simulink model "adc5g_test"  (Simulink 8.0)
settings: Solver=VariableStepDiscrete  SolverMode=SingleTasking  StartTime=0.0  StopTime=10000  RelTol=1e-3
libraries: xbsIndex_r4 x4240, dspsigops x32, casper_library_misc x32, simulink x2, xps_library x1
(part 18 of 29)

== system adc5g_test/hist_0/core_c/subhist0/control ==
  sync : Inport
  bin : Inport  Port=2
  count : Inport  Port=3
  Constant1 : xbsIndex_r4/Constant  const=0  gui_display_data_type=Fixed-point  arith_type=Unsigned  n_bits=46  bin_pt=0  preci_type=Single  exp_width=8  frac_width=24  explicit_period=on  period=1  equ=P=C  opselect=C  inp2=PCIN>>17  opr=+  inp1=P  carry=CIN  dbl_ovrd=off  block_type=constant
  Counter : xbsIndex_r4/Counter  cnt_type=Count Limited  cnt_to=255  operation=Up  start_count=0  cnt_by_val=1  arith_type=Unsigned  n_bits=8  bin_pt=0  load_pin=off  rst=on  en=off  explicit_period=on  period=1  dbl_ovrd=off  use_behavioral_HDL=off  implementation=Fabric  block_type=counter
  Delay17 : xbsIndex_r4/Delay  rst=off  infoeditControl=Selection of Reset will increase slice count due to use of real FFs and instead of SRLs  en=off  latency=7  dbl_ovrd=off  reg_retiming=off  block_type=delay
  Delay2 : xbsIndex_r4/Delay  rst=off  infoeditControl=Selection of Reset will increase slice count due to use of real FFs and instead of SRLs  en=off  latency=7  dbl_ovrd=off  reg_retiming=off  block_type=delay
  Delay3 : xbsIndex_r4/Delay  rst=off  infoeditControl=Selection of Reset will increase slice count due to use of real FFs and instead of SRLs  en=off  latency=7  dbl_ovrd=off  reg_retiming=off  block_type=delay
  Delay5 : xbsIndex_r4/Delay  rst=off  infoeditControl=Selection of Reset will increase slice count due to use of real FFs and instead of SRLs  en=off  latency=1  dbl_ovrd=off  reg_retiming=off  block_type=delay
  Inverter1 : xbsIndex_r4/Inverter  en=off  latency=0  dbl_ovrd=off  block_type=inv
  Logical : xbsIndex_r4/Logical  logical_function=AND  inputs=2  en=off  latency=0  precision=Full  arith_type=Unsigned  n_bits=16  bin_pt=0  align_bp=on  dbl_ovrd=off  block_type=logical
  Mux2 : xbsIndex_r4/Mux  inputs=2  en=off  latency=0  precision=Full  arith_type=Unsigned  n_bits=16  bin_pt=14  quantization=Truncate  overflow=Wrap  dbl_ovrd=off  block_type=mux
  edge_detect : Subsystem  AncestorBlock=casper_library_misc/edge_detect  mask(edge_detect){edge=Rising, polarity=Active High, x_in=[0 0.08 0.08 0.16 0.16 0.24 0.24 0.32 0.32 0.4], y_in=[-1 -1  1  1  1  1  1  1  1  1], x_out=[0.6 0.68 0.68 0.76 0.76 0.84 0.84 0.92 0.92 1], y_out=[-1 -1  1  1 -1 -1 -1 -1 -1 -1]}
      mask prompts: edge "Edge Type"; polarity "Output Polarity"; x_in "Input X Positions"; y_in "Input Y Positions"; x_out "Output X Positions"; y_out "Output Y Positions"
      mask help: Output is active for one-cycle after each edge of the specified type (i.e. rising, falling or both).
      mask init: same as adc5g_test/ramptest_0/posedge
      settings: AttributesFormatString=|
      | rising edges
      | active high
      mask Help: Output is active for one-cycle after each edge of the specified type (i.e. rising, falling, or both).  Latency is zero.
      mask TypeOptions edge: Rising | Falling | Both
      mask TypeOptions polarity: Active High | Active Low
      mask Display:
      | plot(x_in_num,0.5+y_in_num*0.2);
      | plot(x_out_num,0.5+y_out_num*0.2);
  pulse_ext : casper_library_misc/pulse_ext  pulse_len=256
  addra : Outport
  dina : Outport  Port=2
  wea : Outport  Port=3
  addrb : Outport  Port=4
  dinb : Outport  Port=5
  web : Outport  Port=6
  wires:
  sync/1 -> Delay17/1
  Mux2/1 -> addra/1, Delay2/1
  pulse_ext/1 -> Mux2/1, wea/1, Inverter1/1
  Delay5/1 -> Mux2/2
  Delay2/1 -> addrb/1
  Constant1/1 -> dina/1
  Logical/1 -> web/1
  edge_detect/1(out) -> pulse_ext/1, Counter/1
  Counter/1 -> Mux2/3
  Delay3/1 -> Logical/2
  Inverter1/1 -> Logical/1, Delay3/1
  Delay17/1 -> edge_detect/1(in)
  bin/1 -> Delay5/1
  count/1 -> dinb/1

== system adc5g_test/hist_0/core_c/subhist0/precounter ==
  din : Inport
  Constant3 : xbsIndex_r4/Constant  const=1  gui_display_data_type=Fixed-point  arith_type=Unsigned  n_bits=1  bin_pt=0  preci_type=Single  exp_width=8  frac_width=24  explicit_period=on  period=1  equ=P=C  opselect=C  inp2=PCIN>>17  opr=+  inp1=P  carry=CIN  dbl_ovrd=off  block_type=constant
  Delay{1..7} (7 alike) : xbsIndex_r4/Delay  rst=off  infoeditControl=Selection of Reset will increase slice count due to use of real FFs and instead of SRLs  en=off  latency=1  dbl_ovrd=off  reg_retiming=off  block_type=delay
  Reinterpret{,1..6} (7 alike) : xbsIndex_r4/Reinterpret  force_arith_type=on  arith_type=Unsigned  force_bin_pt=off  bin_pt=0  block_type=reinterpret
  Relational{1..7} (7 alike) : xbsIndex_r4/Relational  mode=a=b  en=off  latency=0  dbl_ovrd=off  block_type=relational
  cnt{1..7} (7 alike) : xbsIndex_r4/AddSub  mode=Addition  use_carryin=off  use_carryout=off  en=off  latency=1  precision=Full  arith_type=Unsigned  n_bits=64  bin_pt=0  quantization=Truncate  overflow=Saturate  dbl_ovrd=off  use_behavioral_HDL=off  hw_selection=Fabric  pipelined=on  block_type=addsub
  sum : Outport
  wires:
  cnt1/1 -> cnt3/2
  Constant3/1 -> cnt2/1
  Relational1/1 -> Reinterpret/1
  Relational3/1 -> Reinterpret2/1
  Relational2/1 -> Reinterpret1/1
  cnt2/1 -> cnt3/1
  Reinterpret/1 -> cnt2/2
  Reinterpret2/1 -> cnt1/2
  Delay4/1 -> Relational3/1, Delay6/1
  Reinterpret1/1 -> cnt1/1
  Delay6/1 -> Relational2/1, Delay7/1
  Delay7/1 -> Relational1/1
  cnt5/1 -> cnt6/2
  Relational4/1 -> Reinterpret3/1
  Relational6/1 -> Reinterpret5/1
  Relational5/1 -> Reinterpret4/1
  cnt4/1 -> cnt6/1
  Reinterpret3/1 -> cnt4/2
  Reinterpret5/1 -> cnt5/2
  Reinterpret4/1 -> cnt5/1
  Relational7/1 -> Reinterpret6/1
  Reinterpret6/1 -> cnt4/1
  din/1 -> Relational5/2, Relational4/2, Relational7/2, Relational3/2, Relational2/2, Relational1/2, Relational6/2, Delay5/1
  Delay1/1 -> Relational7/1, Delay4/1
  Delay2/1 -> Relational4/1, Delay1/1
  Delay3/1 -> Relational5/1, Delay2/1
  Delay5/1 -> Relational6/1, Delay3/1
  cnt3/1 -> cnt7/1
  cnt6/1 -> cnt7/2
  cnt7/1 -> sum/1

== system adc5g_test/hist_0/core_c/subhist1/control ==
  sync : Inport
  bin : Inport  Port=2
  count : Inport  Port=3
  Constant1 : xbsIndex_r4/Constant  const=0  gui_display_data_type=Fixed-point  arith_type=Unsigned  n_bits=46  bin_pt=0  preci_type=Single  exp_width=8  frac_width=24  explicit_period=on  period=1  equ=P=C  opselect=C  inp2=PCIN>>17  opr=+  inp1=P  carry=CIN  dbl_ovrd=off  block_type=constant
  Counter : xbsIndex_r4/Counter  cnt_type=Count Limited  cnt_to=255  operation=Up  start_count=0  cnt_by_val=1  arith_type=Unsigned  n_bits=8  bin_pt=0  load_pin=off  rst=on  en=off  explicit_period=on  period=1  dbl_ovrd=off  use_behavioral_HDL=off  implementation=Fabric  block_type=counter
  Delay17 : xbsIndex_r4/Delay  rst=off  infoeditControl=Selection of Reset will increase slice count due to use of real FFs and instead of SRLs  en=off  latency=7  dbl_ovrd=off  reg_retiming=off  block_type=delay
  Delay2 : xbsIndex_r4/Delay  rst=off  infoeditControl=Selection of Reset will increase slice count due to use of real FFs and instead of SRLs  en=off  latency=7  dbl_ovrd=off  reg_retiming=off  block_type=delay
  Delay3 : xbsIndex_r4/Delay  rst=off  infoeditControl=Selection of Reset will increase slice count due to use of real FFs and instead of SRLs  en=off  latency=7  dbl_ovrd=off  reg_retiming=off  block_type=delay
  Delay5 : xbsIndex_r4/Delay  rst=off  infoeditControl=Selection of Reset will increase slice count due to use of real FFs and instead of SRLs  en=off  latency=1  dbl_ovrd=off  reg_retiming=off  block_type=delay
  Inverter1 : xbsIndex_r4/Inverter  en=off  latency=0  dbl_ovrd=off  block_type=inv
  Logical : xbsIndex_r4/Logical  logical_function=AND  inputs=2  en=off  latency=0  precision=Full  arith_type=Unsigned  n_bits=16  bin_pt=0  align_bp=on  dbl_ovrd=off  block_type=logical
  Mux2 : xbsIndex_r4/Mux  inputs=2  en=off  latency=0  precision=Full  arith_type=Unsigned  n_bits=16  bin_pt=14  quantization=Truncate  overflow=Wrap  dbl_ovrd=off  block_type=mux
  edge_detect : Subsystem  AncestorBlock=casper_library_misc/edge_detect  mask(edge_detect){edge=Rising, polarity=Active High, x_in=[0 0.08 0.08 0.16 0.16 0.24 0.24 0.32 0.32 0.4], y_in=[-1 -1  1  1  1  1  1  1  1  1], x_out=[0.6 0.68 0.68 0.76 0.76 0.84 0.84 0.92 0.92 1], y_out=[-1 -1  1  1 -1 -1 -1 -1 -1 -1]}
      mask prompts: edge "Edge Type"; polarity "Output Polarity"; x_in "Input X Positions"; y_in "Input Y Positions"; x_out "Output X Positions"; y_out "Output Y Positions"
      mask help: Output is active for one-cycle after each edge of the specified type (i.e. rising, falling or both).
      mask init: same as adc5g_test/ramptest_0/posedge
      settings: AttributesFormatString=|
      | rising edges
      | active high
      mask Help: Output is active for one-cycle after each edge of the specified type (i.e. rising, falling, or both).  Latency is zero.
      mask TypeOptions edge: Rising | Falling | Both
      mask TypeOptions polarity: Active High | Active Low
      mask Display:
      | plot(x_in_num,0.5+y_in_num*0.2);
      | plot(x_out_num,0.5+y_out_num*0.2);
  pulse_ext : casper_library_misc/pulse_ext  pulse_len=256
  addra : Outport
  dina : Outport  Port=2
  wea : Outport  Port=3
  addrb : Outport  Port=4
  dinb : Outport  Port=5
  web : Outport  Port=6
  wires:
  count/1 -> dinb/1
  bin/1 -> Delay5/1
  Delay17/1 -> edge_detect/1(in)
  Inverter1/1 -> Delay3/1, Logical/1
  Delay3/1 -> Logical/2
  Counter/1 -> Mux2/3
  edge_detect/1(out) -> Counter/1, pulse_ext/1
  Logical/1 -> web/1
  Constant1/1 -> dina/1
  Delay2/1 -> addrb/1
  Delay5/1 -> Mux2/2
  pulse_ext/1 -> Inverter1/1, wea/1, Mux2/1
  Mux2/1 -> Delay2/1, addra/1
  sync/1 -> Delay17/1

== system adc5g_test/hist_0/core_c/subhist1/precounter ==
  din : Inport
  Constant3 : xbsIndex_r4/Constant  const=1  gui_display_data_type=Fixed-point  arith_type=Unsigned  n_bits=1  bin_pt=0  preci_type=Single  exp_width=8  frac_width=24  explicit_period=on  period=1  equ=P=C  opselect=C  inp2=PCIN>>17  opr=+  inp1=P  carry=CIN  dbl_ovrd=off  block_type=constant
  Delay{1..7} (7 alike) : xbsIndex_r4/Delay  rst=off  infoeditControl=Selection of Reset will increase slice count due to use of real FFs and instead of SRLs  en=off  latency=1  dbl_ovrd=off  reg_retiming=off  block_type=delay
  Reinterpret{,1..6} (7 alike) : xbsIndex_r4/Reinterpret  force_arith_type=on  arith_type=Unsigned  force_bin_pt=off  bin_pt=0  block_type=reinterpret
  Relational{1..7} (7 alike) : xbsIndex_r4/Relational  mode=a=b  en=off  latency=0  dbl_ovrd=off  block_type=relational
  cnt{1..7} (7 alike) : xbsIndex_r4/AddSub  mode=Addition  use_carryin=off  use_carryout=off  en=off  latency=1  precision=Full  arith_type=Unsigned  n_bits=64  bin_pt=0  quantization=Truncate  overflow=Saturate  dbl_ovrd=off  use_behavioral_HDL=off  hw_selection=Fabric  pipelined=on  block_type=addsub
  sum : Outport
  wires:
  cnt7/1 -> sum/1
  cnt6/1 -> cnt7/2
  cnt3/1 -> cnt7/1
  Delay5/1 -> Delay3/1, Relational6/1
  Delay3/1 -> Delay2/1, Relational5/1
  Delay2/1 -> Delay1/1, Relational4/1
  Delay1/1 -> Delay4/1, Relational7/1
  din/1 -> Delay5/1, Relational6/2, Relational1/2, Relational2/2, Relational3/2, Relational7/2, Relational4/2, Relational5/2
  Reinterpret6/1 -> cnt4/1
  Relational7/1 -> Reinterpret6/1
  Reinterpret4/1 -> cnt5/1
  Reinterpret5/1 -> cnt5/2
  Reinterpret3/1 -> cnt4/2
  cnt4/1 -> cnt6/1
  Relational5/1 -> Reinterpret4/1
  Relational6/1 -> Reinterpret5/1
  Relational4/1 -> Reinterpret3/1
  cnt5/1 -> cnt6/2
  Delay7/1 -> Relational1/1
  Delay6/1 -> Delay7/1, Relational2/1
  Reinterpret1/1 -> cnt1/1
  Delay4/1 -> Delay6/1, Relational3/1
  Reinterpret2/1 -> cnt1/2
  Reinterpret/1 -> cnt2/2
  cnt2/1 -> cnt3/1
  Relational2/1 -> Reinterpret1/1
  Relational3/1 -> Reinterpret2/1
  Relational1/1 -> Reinterpret/1
  Constant3/1 -> cnt2/1
  cnt1/1 -> cnt3/2

== system adc5g_test/hist_0/core_c/subhist2/control ==
  sync : Inport
  bin : Inport  Port=2
  count : Inport  Port=3
  Constant1 : xbsIndex_r4/Constant  const=0  gui_display_data_type=Fixed-point  arith_type=Unsigned  n_bits=46  bin_pt=0  preci_type=Single  exp_width=8  frac_width=24  explicit_period=on  period=1  equ=P=C  opselect=C  inp2=PCIN>>17  opr=+  inp1=P  carry=CIN  dbl_ovrd=off  block_type=constant
  Counter : xbsIndex_r4/Counter  cnt_type=Count Limited  cnt_to=255  operation=Up  start_count=0  cnt_by_val=1  arith_type=Unsigned  n_bits=8  bin_pt=0  load_pin=off  rst=on  en=off  explicit_period=on  period=1  dbl_ovrd=off  use_behavioral_HDL=off  implementation=Fabric  block_type=counter
  Delay17 : xbsIndex_r4/Delay  rst=off  infoeditControl=Selection of Reset will increase slice count due to use of real FFs and instead of SRLs  en=off  latency=7  dbl_ovrd=off  reg_retiming=off  block_type=delay
  Delay2 : xbsIndex_r4/Delay  rst=off  infoeditControl=Selection of Reset will increase slice count due to use of real FFs and instead of SRLs  en=off  latency=7  dbl_ovrd=off  reg_retiming=off  block_type=delay
  Delay3 : xbsIndex_r4/Delay  rst=off  infoeditControl=Selection of Reset will increase slice count due to use of real FFs and instead of SRLs  en=off  latency=7  dbl_ovrd=off  reg_retiming=off  block_type=delay
  Delay5 : xbsIndex_r4/Delay  rst=off  infoeditControl=Selection of Reset will increase slice count due to use of real FFs and instead of SRLs  en=off  latency=1  dbl_ovrd=off  reg_retiming=off  block_type=delay
  Inverter1 : xbsIndex_r4/Inverter  en=off  latency=0  dbl_ovrd=off  block_type=inv
  Logical : xbsIndex_r4/Logical  logical_function=AND  inputs=2  en=off  latency=0  precision=Full  arith_type=Unsigned  n_bits=16  bin_pt=0  align_bp=on  dbl_ovrd=off  block_type=logical
  Mux2 : xbsIndex_r4/Mux  inputs=2  en=off  latency=0  precision=Full  arith_type=Unsigned  n_bits=16  bin_pt=14  quantization=Truncate  overflow=Wrap  dbl_ovrd=off  block_type=mux
  edge_detect : Subsystem  AncestorBlock=casper_library_misc/edge_detect  mask(edge_detect){edge=Rising, polarity=Active High, x_in=[0 0.08 0.08 0.16 0.16 0.24 0.24 0.32 0.32 0.4], y_in=[-1 -1  1  1  1  1  1  1  1  1], x_out=[0.6 0.68 0.68 0.76 0.76 0.84 0.84 0.92 0.92 1], y_out=[-1 -1  1  1 -1 -1 -1 -1 -1 -1]}
      mask prompts: edge "Edge Type"; polarity "Output Polarity"; x_in "Input X Positions"; y_in "Input Y Positions"; x_out "Output X Positions"; y_out "Output Y Positions"
      mask help: Output is active for one-cycle after each edge of the specified type (i.e. rising, falling or both).
      mask init: same as adc5g_test/ramptest_0/posedge
      settings: AttributesFormatString=|
      | rising edges
      | active high
      mask Help: Output is active for one-cycle after each edge of the specified type (i.e. rising, falling, or both).  Latency is zero.
      mask TypeOptions edge: Rising | Falling | Both
      mask TypeOptions polarity: Active High | Active Low
      mask Display:
      | plot(x_in_num,0.5+y_in_num*0.2);
      | plot(x_out_num,0.5+y_out_num*0.2);
  pulse_ext : casper_library_misc/pulse_ext  pulse_len=256
  addra : Outport
  dina : Outport  Port=2
  wea : Outport  Port=3
  addrb : Outport  Port=4
  dinb : Outport  Port=5
  web : Outport  Port=6
  wires:
  sync/1 -> Delay17/1
  Mux2/1 -> addra/1, Delay2/1
  pulse_ext/1 -> Mux2/1, wea/1, Inverter1/1
  Delay5/1 -> Mux2/2
  Delay2/1 -> addrb/1
  Constant1/1 -> dina/1
  Logical/1 -> web/1
  edge_detect/1(out) -> pulse_ext/1, Counter/1
  Counter/1 -> Mux2/3
  Delay3/1 -> Logical/2
  Inverter1/1 -> Logical/1, Delay3/1
  Delay17/1 -> edge_detect/1(in)
  bin/1 -> Delay5/1
  count/1 -> dinb/1

== system adc5g_test/hist_0/core_c/subhist2/precounter ==
  din : Inport
  Constant3 : xbsIndex_r4/Constant  const=1  gui_display_data_type=Fixed-point  arith_type=Unsigned  n_bits=1  bin_pt=0  preci_type=Single  exp_width=8  frac_width=24  explicit_period=on  period=1  equ=P=C  opselect=C  inp2=PCIN>>17  opr=+  inp1=P  carry=CIN  dbl_ovrd=off  block_type=constant
  Delay{1..7} (7 alike) : xbsIndex_r4/Delay  rst=off  infoeditControl=Selection of Reset will increase slice count due to use of real FFs and instead of SRLs  en=off  latency=1  dbl_ovrd=off  reg_retiming=off  block_type=delay
  Reinterpret{,1..6} (7 alike) : xbsIndex_r4/Reinterpret  force_arith_type=on  arith_type=Unsigned  force_bin_pt=off  bin_pt=0  block_type=reinterpret
  Relational{1..7} (7 alike) : xbsIndex_r4/Relational  mode=a=b  en=off  latency=0  dbl_ovrd=off  block_type=relational
  cnt{1..7} (7 alike) : xbsIndex_r4/AddSub  mode=Addition  use_carryin=off  use_carryout=off  en=off  latency=1  precision=Full  arith_type=Unsigned  n_bits=64  bin_pt=0  quantization=Truncate  overflow=Saturate  dbl_ovrd=off  use_behavioral_HDL=off  hw_selection=Fabric  pipelined=on  block_type=addsub
  sum : Outport
  wires:
  cnt1/1 -> cnt3/2
  Constant3/1 -> cnt2/1
  Relational1/1 -> Reinterpret/1
  Relational3/1 -> Reinterpret2/1
  Relational2/1 -> Reinterpret1/1
  cnt2/1 -> cnt3/1
  Reinterpret/1 -> cnt2/2
  Reinterpret2/1 -> cnt1/2
  Delay4/1 -> Relational3/1, Delay6/1
  Reinterpret1/1 -> cnt1/1
  Delay6/1 -> Relational2/1, Delay7/1
  Delay7/1 -> Relational1/1
  cnt5/1 -> cnt6/2
  Relational4/1 -> Reinterpret3/1
  Relational6/1 -> Reinterpret5/1
  Relational5/1 -> Reinterpret4/1
  cnt4/1 -> cnt6/1
  Reinterpret3/1 -> cnt4/2
  Reinterpret5/1 -> cnt5/2
  Reinterpret4/1 -> cnt5/1
  Relational7/1 -> Reinterpret6/1
  Reinterpret6/1 -> cnt4/1
  din/1 -> Relational5/2, Relational4/2, Relational7/2, Relational3/2, Relational2/2, Relational1/2, Relational6/2, Delay5/1
  Delay1/1 -> Relational7/1, Delay4/1
  Delay2/1 -> Relational4/1, Delay1/1
  Delay3/1 -> Relational5/1, Delay2/1
  Delay5/1 -> Relational6/1, Delay3/1
  cnt3/1 -> cnt7/1
  cnt6/1 -> cnt7/2
  cnt7/1 -> sum/1

== system adc5g_test/hist_0/core_c/subhist3/control ==
  sync : Inport
  bin : Inport  Port=2
  count : Inport  Port=3
  Constant1 : xbsIndex_r4/Constant  const=0  gui_display_data_type=Fixed-point  arith_type=Unsigned  n_bits=46  bin_pt=0  preci_type=Single  exp_width=8  frac_width=24  explicit_period=on  period=1  equ=P=C  opselect=C  inp2=PCIN>>17  opr=+  inp1=P  carry=CIN  dbl_ovrd=off  block_type=constant
  Counter : xbsIndex_r4/Counter  cnt_type=Count Limited  cnt_to=255  operation=Up  start_count=0  cnt_by_val=1  arith_type=Unsigned  n_bits=8  bin_pt=0  load_pin=off  rst=on  en=off  explicit_period=on  period=1  dbl_ovrd=off  use_behavioral_HDL=off  implementation=Fabric  block_type=counter
  Delay17 : xbsIndex_r4/Delay  rst=off  infoeditControl=Selection of Reset will increase slice count due to use of real FFs and instead of SRLs  en=off  latency=7  dbl_ovrd=off  reg_retiming=off  block_type=delay
  Delay2 : xbsIndex_r4/Delay  rst=off  infoeditControl=Selection of Reset will increase slice count due to use of real FFs and instead of SRLs  en=off  latency=7  dbl_ovrd=off  reg_retiming=off  block_type=delay
  Delay3 : xbsIndex_r4/Delay  rst=off  infoeditControl=Selection of Reset will increase slice count due to use of real FFs and instead of SRLs  en=off  latency=7  dbl_ovrd=off  reg_retiming=off  block_type=delay
  Delay5 : xbsIndex_r4/Delay  rst=off  infoeditControl=Selection of Reset will increase slice count due to use of real FFs and instead of SRLs  en=off  latency=1  dbl_ovrd=off  reg_retiming=off  block_type=delay
  Inverter1 : xbsIndex_r4/Inverter  en=off  latency=0  dbl_ovrd=off  block_type=inv
  Logical : xbsIndex_r4/Logical  logical_function=AND  inputs=2  en=off  latency=0  precision=Full  arith_type=Unsigned  n_bits=16  bin_pt=0  align_bp=on  dbl_ovrd=off  block_type=logical
  Mux2 : xbsIndex_r4/Mux  inputs=2  en=off  latency=0  precision=Full  arith_type=Unsigned  n_bits=16  bin_pt=14  quantization=Truncate  overflow=Wrap  dbl_ovrd=off  block_type=mux
  edge_detect : Subsystem  AncestorBlock=casper_library_misc/edge_detect  mask(edge_detect){edge=Rising, polarity=Active High, x_in=[0 0.08 0.08 0.16 0.16 0.24 0.24 0.32 0.32 0.4], y_in=[-1 -1  1  1  1  1  1  1  1  1], x_out=[0.6 0.68 0.68 0.76 0.76 0.84 0.84 0.92 0.92 1], y_out=[-1 -1  1  1 -1 -1 -1 -1 -1 -1]}
      mask prompts: edge "Edge Type"; polarity "Output Polarity"; x_in "Input X Positions"; y_in "Input Y Positions"; x_out "Output X Positions"; y_out "Output Y Positions"
      mask help: Output is active for one-cycle after each edge of the specified type (i.e. rising, falling or both).
      mask init: same as adc5g_test/ramptest_0/posedge
      settings: AttributesFormatString=|
      | rising edges
      | active high
      mask Help: Output is active for one-cycle after each edge of the specified type (i.e. rising, falling, or both).  Latency is zero.
      mask TypeOptions edge: Rising | Falling | Both
      mask TypeOptions polarity: Active High | Active Low
      mask Display:
      | plot(x_in_num,0.5+y_in_num*0.2);
      | plot(x_out_num,0.5+y_out_num*0.2);
  pulse_ext : casper_library_misc/pulse_ext  pulse_len=256
  addra : Outport
  dina : Outport  Port=2
  wea : Outport  Port=3
  addrb : Outport  Port=4
  dinb : Outport  Port=5
  web : Outport  Port=6
  wires:
  count/1 -> dinb/1
  bin/1 -> Delay5/1
  Delay17/1 -> edge_detect/1(in)
  Inverter1/1 -> Delay3/1, Logical/1
  Delay3/1 -> Logical/2
  Counter/1 -> Mux2/3
  edge_detect/1(out) -> Counter/1, pulse_ext/1
  Logical/1 -> web/1
  Constant1/1 -> dina/1
  Delay2/1 -> addrb/1
  Delay5/1 -> Mux2/2
  pulse_ext/1 -> Inverter1/1, wea/1, Mux2/1
  Mux2/1 -> Delay2/1, addra/1
  sync/1 -> Delay17/1

== system adc5g_test/hist_0/core_c/subhist3/precounter ==
  din : Inport
  Constant3 : xbsIndex_r4/Constant  const=1  gui_display_data_type=Fixed-point  arith_type=Unsigned  n_bits=1  bin_pt=0  preci_type=Single  exp_width=8  frac_width=24  explicit_period=on  period=1  equ=P=C  opselect=C  inp2=PCIN>>17  opr=+  inp1=P  carry=CIN  dbl_ovrd=off  block_type=constant
  Delay{1..7} (7 alike) : xbsIndex_r4/Delay  rst=off  infoeditControl=Selection of Reset will increase slice count due to use of real FFs and instead of SRLs  en=off  latency=1  dbl_ovrd=off  reg_retiming=off  block_type=delay
  Reinterpret{,1..6} (7 alike) : xbsIndex_r4/Reinterpret  force_arith_type=on  arith_type=Unsigned  force_bin_pt=off  bin_pt=0  block_type=reinterpret
  Relational{1..7} (7 alike) : xbsIndex_r4/Relational  mode=a=b  en=off  latency=0  dbl_ovrd=off  block_type=relational
  cnt{1..7} (7 alike) : xbsIndex_r4/AddSub  mode=Addition  use_carryin=off  use_carryout=off  en=off  latency=1  precision=Full  arith_type=Unsigned  n_bits=64  bin_pt=0  quantization=Truncate  overflow=Saturate  dbl_ovrd=off  use_behavioral_HDL=off  hw_selection=Fabric  pipelined=on  block_type=addsub
  sum : Outport
  wires:
  cnt7/1 -> sum/1
  cnt6/1 -> cnt7/2
  cnt3/1 -> cnt7/1
  Delay5/1 -> Delay3/1, Relational6/1
  Delay3/1 -> Delay2/1, Relational5/1
  Delay2/1 -> Delay1/1, Relational4/1
  Delay1/1 -> Delay4/1, Relational7/1
  din/1 -> Delay5/1, Relational6/2, Relational1/2, Relational2/2, Relational3/2, Relational7/2, Relational4/2, Relational5/2
  Reinterpret6/1 -> cnt4/1
  Relational7/1 -> Reinterpret6/1
  Reinterpret4/1 -> cnt5/1
  Reinterpret5/1 -> cnt5/2
  Reinterpret3/1 -> cnt4/2
  cnt4/1 -> cnt6/1
  Relational5/1 -> Reinterpret4/1
  Relational6/1 -> Reinterpret5/1
  Relational4/1 -> Reinterpret3/1
  cnt5/1 -> cnt6/2
  Delay7/1 -> Relational1/1
  Delay6/1 -> Delay7/1, Relational2/1
  Reinterpret1/1 -> cnt1/1
  Delay4/1 -> Delay6/1, Relational3/1
  Reinterpret2/1 -> cnt1/2
  Reinterpret/1 -> cnt2/2
  cnt2/1 -> cnt3/1
  Relational2/1 -> Reinterpret1/1
  Relational3/1 -> Reinterpret2/1
  Relational1/1 -> Reinterpret/1
  Constant3/1 -> cnt2/1
  cnt1/1 -> cnt3/2

== system adc5g_test/hist_0/core_d/subhist0/control ==
  sync : Inport
  bin : Inport  Port=2
  count : Inport  Port=3
  Constant1 : xbsIndex_r4/Constant  const=0  gui_display_data_type=Fixed-point  arith_type=Unsigned  n_bits=46  bin_pt=0  preci_type=Single  exp_width=8  frac_width=24  explicit_period=on  period=1  equ=P=C  opselect=C  inp2=PCIN>>17  opr=+  inp1=P  carry=CIN  dbl_ovrd=off  block_type=constant
  Counter : xbsIndex_r4/Counter  cnt_type=Count Limited  cnt_to=255  operation=Up  start_count=0  cnt_by_val=1  arith_type=Unsigned  n_bits=8  bin_pt=0  load_pin=off  rst=on  en=off  explicit_period=on  period=1  dbl_ovrd=off  use_behavioral_HDL=off  implementation=Fabric  block_type=counter
  Delay17 : xbsIndex_r4/Delay  rst=off  infoeditControl=Selection of Reset will increase slice count due to use of real FFs and instead of SRLs  en=off  latency=7  dbl_ovrd=off  reg_retiming=off  block_type=delay
  Delay2 : xbsIndex_r4/Delay  rst=off  infoeditControl=Selection of Reset will increase slice count due to use of real FFs and instead of SRLs  en=off  latency=7  dbl_ovrd=off  reg_retiming=off  block_type=delay
  Delay3 : xbsIndex_r4/Delay  rst=off  infoeditControl=Selection of Reset will increase slice count due to use of real FFs and instead of SRLs  en=off  latency=7  dbl_ovrd=off  reg_retiming=off  block_type=delay
  Delay5 : xbsIndex_r4/Delay  rst=off  infoeditControl=Selection of Reset will increase slice count due to use of real FFs and instead of SRLs  en=off  latency=1  dbl_ovrd=off  reg_retiming=off  block_type=delay
  Inverter1 : xbsIndex_r4/Inverter  en=off  latency=0  dbl_ovrd=off  block_type=inv
  Logical : xbsIndex_r4/Logical  logical_function=AND  inputs=2  en=off  latency=0  precision=Full  arith_type=Unsigned  n_bits=16  bin_pt=0  align_bp=on  dbl_ovrd=off  block_type=logical
  Mux2 : xbsIndex_r4/Mux  inputs=2  en=off  latency=0  precision=Full  arith_type=Unsigned  n_bits=16  bin_pt=14  quantization=Truncate  overflow=Wrap  dbl_ovrd=off  block_type=mux
  edge_detect : Subsystem  AncestorBlock=casper_library_misc/edge_detect  mask(edge_detect){edge=Rising, polarity=Active High, x_in=[0 0.08 0.08 0.16 0.16 0.24 0.24 0.32 0.32 0.4], y_in=[-1 -1  1  1  1  1  1  1  1  1], x_out=[0.6 0.68 0.68 0.76 0.76 0.84 0.84 0.92 0.92 1], y_out=[-1 -1  1  1 -1 -1 -1 -1 -1 -1]}
      mask prompts: edge "Edge Type"; polarity "Output Polarity"; x_in "Input X Positions"; y_in "Input Y Positions"; x_out "Output X Positions"; y_out "Output Y Positions"
      mask help: Output is active for one-cycle after each edge of the specified type (i.e. rising, falling or both).
      mask init: same as adc5g_test/ramptest_0/posedge
      settings: AttributesFormatString=|
      | rising edges
      | active high
      mask Help: Output is active for one-cycle after each edge of the specified type (i.e. rising, falling, or both).  Latency is zero.
      mask TypeOptions edge: Rising | Falling | Both
      mask TypeOptions polarity: Active High | Active Low
      mask Display:
      | plot(x_in_num,0.5+y_in_num*0.2);
      | plot(x_out_num,0.5+y_out_num*0.2);
  pulse_ext : casper_library_misc/pulse_ext  pulse_len=256
  addra : Outport
  dina : Outport  Port=2
  wea : Outport  Port=3
  addrb : Outport  Port=4
  dinb : Outport  Port=5
  web : Outport  Port=6
  wires:
  count/1 -> dinb/1
  bin/1 -> Delay5/1
  Delay17/1 -> edge_detect/1(in)
  Inverter1/1 -> Delay3/1, Logical/1
  Delay3/1 -> Logical/2
  Counter/1 -> Mux2/3
  edge_detect/1(out) -> Counter/1, pulse_ext/1
  Logical/1 -> web/1
  Constant1/1 -> dina/1
  Delay2/1 -> addrb/1
  Delay5/1 -> Mux2/2
  pulse_ext/1 -> Inverter1/1, wea/1, Mux2/1
  Mux2/1 -> Delay2/1, addra/1
  sync/1 -> Delay17/1

== system adc5g_test/hist_0/core_d/subhist0/precounter ==
  din : Inport
  Constant3 : xbsIndex_r4/Constant  const=1  gui_display_data_type=Fixed-point  arith_type=Unsigned  n_bits=1  bin_pt=0  preci_type=Single  exp_width=8  frac_width=24  explicit_period=on  period=1  equ=P=C  opselect=C  inp2=PCIN>>17  opr=+  inp1=P  carry=CIN  dbl_ovrd=off  block_type=constant
  Delay{1..7} (7 alike) : xbsIndex_r4/Delay  rst=off  infoeditControl=Selection of Reset will increase slice count due to use of real FFs and instead of SRLs  en=off  latency=1  dbl_ovrd=off  reg_retiming=off  block_type=delay
  Reinterpret{,1..6} (7 alike) : xbsIndex_r4/Reinterpret  force_arith_type=on  arith_type=Unsigned  force_bin_pt=off  bin_pt=0  block_type=reinterpret
  Relational{1..7} (7 alike) : xbsIndex_r4/Relational  mode=a=b  en=off  latency=0  dbl_ovrd=off  block_type=relational
  cnt{1..7} (7 alike) : xbsIndex_r4/AddSub  mode=Addition  use_carryin=off  use_carryout=off  en=off  latency=1  precision=Full  arith_type=Unsigned  n_bits=64  bin_pt=0  quantization=Truncate  overflow=Saturate  dbl_ovrd=off  use_behavioral_HDL=off  hw_selection=Fabric  pipelined=on  block_type=addsub
  sum : Outport
  wires:
  cnt7/1 -> sum/1
  cnt6/1 -> cnt7/2
  cnt3/1 -> cnt7/1
  Delay5/1 -> Delay3/1, Relational6/1
  Delay3/1 -> Delay2/1, Relational5/1
  Delay2/1 -> Delay1/1, Relational4/1
  Delay1/1 -> Delay4/1, Relational7/1
  din/1 -> Delay5/1, Relational6/2, Relational1/2, Relational2/2, Relational3/2, Relational7/2, Relational4/2, Relational5/2
  Reinterpret6/1 -> cnt4/1
  Relational7/1 -> Reinterpret6/1
  Reinterpret4/1 -> cnt5/1
  Reinterpret5/1 -> cnt5/2
  Reinterpret3/1 -> cnt4/2
  cnt4/1 -> cnt6/1
  Relational5/1 -> Reinterpret4/1
  Relational6/1 -> Reinterpret5/1
  Relational4/1 -> Reinterpret3/1
  cnt5/1 -> cnt6/2
  Delay7/1 -> Relational1/1
  Delay6/1 -> Delay7/1, Relational2/1
  Reinterpret1/1 -> cnt1/1
  Delay4/1 -> Delay6/1, Relational3/1
  Reinterpret2/1 -> cnt1/2
  Reinterpret/1 -> cnt2/2
  cnt2/1 -> cnt3/1
  Relational2/1 -> Reinterpret1/1
  Relational3/1 -> Reinterpret2/1
  Relational1/1 -> Reinterpret/1
  Constant3/1 -> cnt2/1
  cnt1/1 -> cnt3/2

== system adc5g_test/hist_0/core_d/subhist1/control ==
  sync : Inport
  bin : Inport  Port=2
  count : Inport  Port=3
  Constant1 : xbsIndex_r4/Constant  const=0  gui_display_data_type=Fixed-point  arith_type=Unsigned  n_bits=46  bin_pt=0  preci_type=Single  exp_width=8  frac_width=24  explicit_period=on  period=1  equ=P=C  opselect=C  inp2=PCIN>>17  opr=+  inp1=P  carry=CIN  dbl_ovrd=off  block_type=constant
  Counter : xbsIndex_r4/Counter  cnt_type=Count Limited  cnt_to=255  operation=Up  start_count=0  cnt_by_val=1  arith_type=Unsigned  n_bits=8  bin_pt=0  load_pin=off  rst=on  en=off  explicit_period=on  period=1  dbl_ovrd=off  use_behavioral_HDL=off  implementation=Fabric  block_type=counter
  Delay17 : xbsIndex_r4/Delay  rst=off  infoeditControl=Selection of Reset will increase slice count due to use of real FFs and instead of SRLs  en=off  latency=7  dbl_ovrd=off  reg_retiming=off  block_type=delay
  Delay2 : xbsIndex_r4/Delay  rst=off  infoeditControl=Selection of Reset will increase slice count due to use of real FFs and instead of SRLs  en=off  latency=7  dbl_ovrd=off  reg_retiming=off  block_type=delay
  Delay3 : xbsIndex_r4/Delay  rst=off  infoeditControl=Selection of Reset will increase slice count due to use of real FFs and instead of SRLs  en=off  latency=7  dbl_ovrd=off  reg_retiming=off  block_type=delay
  Delay5 : xbsIndex_r4/Delay  rst=off  infoeditControl=Selection of Reset will increase slice count due to use of real FFs and instead of SRLs  en=off  latency=1  dbl_ovrd=off  reg_retiming=off  block_type=delay
  Inverter1 : xbsIndex_r4/Inverter  en=off  latency=0  dbl_ovrd=off  block_type=inv
  Logical : xbsIndex_r4/Logical  logical_function=AND  inputs=2  en=off  latency=0  precision=Full  arith_type=Unsigned  n_bits=16  bin_pt=0  align_bp=on  dbl_ovrd=off  block_type=logical
  Mux2 : xbsIndex_r4/Mux  inputs=2  en=off  latency=0  precision=Full  arith_type=Unsigned  n_bits=16  bin_pt=14  quantization=Truncate  overflow=Wrap  dbl_ovrd=off  block_type=mux
  edge_detect : Subsystem  AncestorBlock=casper_library_misc/edge_detect  mask(edge_detect){edge=Rising, polarity=Active High, x_in=[0 0.08 0.08 0.16 0.16 0.24 0.24 0.32 0.32 0.4], y_in=[-1 -1  1  1  1  1  1  1  1  1], x_out=[0.6 0.68 0.68 0.76 0.76 0.84 0.84 0.92 0.92 1], y_out=[-1 -1  1  1 -1 -1 -1 -1 -1 -1]}
      mask prompts: edge "Edge Type"; polarity "Output Polarity"; x_in "Input X Positions"; y_in "Input Y Positions"; x_out "Output X Positions"; y_out "Output Y Positions"
      mask help: Output is active for one-cycle after each edge of the specified type (i.e. rising, falling or both).
      mask init: same as adc5g_test/ramptest_0/posedge
      settings: AttributesFormatString=|
      | rising edges
      | active high
      mask Help: Output is active for one-cycle after each edge of the specified type (i.e. rising, falling, or both).  Latency is zero.
      mask TypeOptions edge: Rising | Falling | Both
      mask TypeOptions polarity: Active High | Active Low
      mask Display:
      | plot(x_in_num,0.5+y_in_num*0.2);
      | plot(x_out_num,0.5+y_out_num*0.2);
  pulse_ext : casper_library_misc/pulse_ext  pulse_len=256
  addra : Outport
  dina : Outport  Port=2
  wea : Outport  Port=3
  addrb : Outport  Port=4
  dinb : Outport  Port=5
  web : Outport  Port=6
  wires:
  sync/1 -> Delay17/1
  Mux2/1 -> addra/1, Delay2/1
  pulse_ext/1 -> Mux2/1, wea/1, Inverter1/1
  Delay5/1 -> Mux2/2
  Delay2/1 -> addrb/1
  Constant1/1 -> dina/1
  Logical/1 -> web/1
  edge_detect/1(out) -> pulse_ext/1, Counter/1
  Counter/1 -> Mux2/3
  Delay3/1 -> Logical/2
  Inverter1/1 -> Logical/1, Delay3/1
  Delay17/1 -> edge_detect/1(in)
  bin/1 -> Delay5/1
  count/1 -> dinb/1

== system adc5g_test/hist_0/core_d/subhist1/precounter ==
  din : Inport
  Constant3 : xbsIndex_r4/Constant  const=1  gui_display_data_type=Fixed-point  arith_type=Unsigned  n_bits=1  bin_pt=0  preci_type=Single  exp_width=8  frac_width=24  explicit_period=on  period=1  equ=P=C  opselect=C  inp2=PCIN>>17  opr=+  inp1=P  carry=CIN  dbl_ovrd=off  block_type=constant
  Delay{1..7} (7 alike) : xbsIndex_r4/Delay  rst=off  infoeditControl=Selection of Reset will increase slice count due to use of real FFs and instead of SRLs  en=off  latency=1  dbl_ovrd=off  reg_retiming=off  block_type=delay
  Reinterpret{,1..6} (7 alike) : xbsIndex_r4/Reinterpret  force_arith_type=on  arith_type=Unsigned  force_bin_pt=off  bin_pt=0  block_type=reinterpret
  Relational{1..7} (7 alike) : xbsIndex_r4/Relational  mode=a=b  en=off  latency=0  dbl_ovrd=off  block_type=relational
  cnt{1..7} (7 alike) : xbsIndex_r4/AddSub  mode=Addition  use_carryin=off  use_carryout=off  en=off  latency=1  precision=Full  arith_type=Unsigned  n_bits=64  bin_pt=0  quantization=Truncate  overflow=Saturate  dbl_ovrd=off  use_behavioral_HDL=off  hw_selection=Fabric  pipelined=on  block_type=addsub
  sum : Outport
  wires:
  cnt1/1 -> cnt3/2
  Constant3/1 -> cnt2/1
  Relational1/1 -> Reinterpret/1
  Relational3/1 -> Reinterpret2/1
  Relational2/1 -> Reinterpret1/1
  cnt2/1 -> cnt3/1
  Reinterpret/1 -> cnt2/2
  Reinterpret2/1 -> cnt1/2
  Delay4/1 -> Relational3/1, Delay6/1
  Reinterpret1/1 -> cnt1/1
  Delay6/1 -> Relational2/1, Delay7/1
  Delay7/1 -> Relational1/1
  cnt5/1 -> cnt6/2
  Relational4/1 -> Reinterpret3/1
  Relational6/1 -> Reinterpret5/1
  Relational5/1 -> Reinterpret4/1
  cnt4/1 -> cnt6/1
  Reinterpret3/1 -> cnt4/2
  Reinterpret5/1 -> cnt5/2
  Reinterpret4/1 -> cnt5/1
  Relational7/1 -> Reinterpret6/1
  Reinterpret6/1 -> cnt4/1
  din/1 -> Relational5/2, Relational4/2, Relational7/2, Relational3/2, Relational2/2, Relational1/2, Relational6/2, Delay5/1
  Delay1/1 -> Relational7/1, Delay4/1
  Delay2/1 -> Relational4/1, Delay1/1
  Delay3/1 -> Relational5/1, Delay2/1
  Delay5/1 -> Relational6/1, Delay3/1
  cnt3/1 -> cnt7/1
  cnt6/1 -> cnt7/2
  cnt7/1 -> sum/1

== system adc5g_test/hist_0/core_d/subhist2/control ==
  sync : Inport
  bin : Inport  Port=2
  count : Inport  Port=3
  Constant1 : xbsIndex_r4/Constant  const=0  gui_display_data_type=Fixed-point  arith_type=Unsigned  n_bits=46  bin_pt=0  preci_type=Single  exp_width=8  frac_width=24  explicit_period=on  period=1  equ=P=C  opselect=C  inp2=PCIN>>17  opr=+  inp1=P  carry=CIN  dbl_ovrd=off  block_type=constant
  Counter : xbsIndex_r4/Counter  cnt_type=Count Limited  cnt_to=255  operation=Up  start_count=0  cnt_by_val=1  arith_type=Unsigned  n_bits=8  bin_pt=0  load_pin=off  rst=on  en=off  explicit_period=on  period=1  dbl_ovrd=off  use_behavioral_HDL=off  implementation=Fabric  block_type=counter
  Delay17 : xbsIndex_r4/Delay  rst=off  infoeditControl=Selection of Reset will increase slice count due to use of real FFs and instead of SRLs  en=off  latency=7  dbl_ovrd=off  reg_retiming=off  block_type=delay
  Delay2 : xbsIndex_r4/Delay  rst=off  infoeditControl=Selection of Reset will increase slice count due to use of real FFs and instead of SRLs  en=off  latency=7  dbl_ovrd=off  reg_retiming=off  block_type=delay
  Delay3 : xbsIndex_r4/Delay  rst=off  infoeditControl=Selection of Reset will increase slice count due to use of real FFs and instead of SRLs  en=off  latency=7  dbl_ovrd=off  reg_retiming=off  block_type=delay
  Delay5 : xbsIndex_r4/Delay  rst=off  infoeditControl=Selection of Reset will increase slice count due to use of real FFs and instead of SRLs  en=off  latency=1  dbl_ovrd=off  reg_retiming=off  block_type=delay
  Inverter1 : xbsIndex_r4/Inverter  en=off  latency=0  dbl_ovrd=off  block_type=inv
  Logical : xbsIndex_r4/Logical  logical_function=AND  inputs=2  en=off  latency=0  precision=Full  arith_type=Unsigned  n_bits=16  bin_pt=0  align_bp=on  dbl_ovrd=off  block_type=logical
  Mux2 : xbsIndex_r4/Mux  inputs=2  en=off  latency=0  precision=Full  arith_type=Unsigned  n_bits=16  bin_pt=14  quantization=Truncate  overflow=Wrap  dbl_ovrd=off  block_type=mux
  edge_detect : Subsystem  AncestorBlock=casper_library_misc/edge_detect  mask(edge_detect){edge=Rising, polarity=Active High, x_in=[0 0.08 0.08 0.16 0.16 0.24 0.24 0.32 0.32 0.4], y_in=[-1 -1  1  1  1  1  1  1  1  1], x_out=[0.6 0.68 0.68 0.76 0.76 0.84 0.84 0.92 0.92 1], y_out=[-1 -1  1  1 -1 -1 -1 -1 -1 -1]}
      mask prompts: edge "Edge Type"; polarity "Output Polarity"; x_in "Input X Positions"; y_in "Input Y Positions"; x_out "Output X Positions"; y_out "Output Y Positions"
      mask help: Output is active for one-cycle after each edge of the specified type (i.e. rising, falling or both).
      mask init: same as adc5g_test/ramptest_0/posedge
      settings: AttributesFormatString=|
      | rising edges
      | active high
      mask Help: Output is active for one-cycle after each edge of the specified type (i.e. rising, falling, or both).  Latency is zero.
      mask TypeOptions edge: Rising | Falling | Both
      mask TypeOptions polarity: Active High | Active Low
      mask Display:
      | plot(x_in_num,0.5+y_in_num*0.2);
      | plot(x_out_num,0.5+y_out_num*0.2);
  pulse_ext : casper_library_misc/pulse_ext  pulse_len=256
  addra : Outport
  dina : Outport  Port=2
  wea : Outport  Port=3
  addrb : Outport  Port=4
  dinb : Outport  Port=5
  web : Outport  Port=6
  wires:
  count/1 -> dinb/1
  bin/1 -> Delay5/1
  Delay17/1 -> edge_detect/1(in)
  Inverter1/1 -> Delay3/1, Logical/1
  Delay3/1 -> Logical/2
  Counter/1 -> Mux2/3
  edge_detect/1(out) -> Counter/1, pulse_ext/1
  Logical/1 -> web/1
  Constant1/1 -> dina/1
  Delay2/1 -> addrb/1
  Delay5/1 -> Mux2/2
  pulse_ext/1 -> Inverter1/1, wea/1, Mux2/1
  Mux2/1 -> Delay2/1, addra/1
  sync/1 -> Delay17/1

== system adc5g_test/hist_0/core_d/subhist2/precounter ==
  din : Inport
  Constant3 : xbsIndex_r4/Constant  const=1  gui_display_data_type=Fixed-point  arith_type=Unsigned  n_bits=1  bin_pt=0  preci_type=Single  exp_width=8  frac_width=24  explicit_period=on  period=1  equ=P=C  opselect=C  inp2=PCIN>>17  opr=+  inp1=P  carry=CIN  dbl_ovrd=off  block_type=constant
  Delay{1..7} (7 alike) : xbsIndex_r4/Delay  rst=off  infoeditControl=Selection of Reset will increase slice count due to use of real FFs and instead of SRLs  en=off  latency=1  dbl_ovrd=off  reg_retiming=off  block_type=delay
  Reinterpret{,1..6} (7 alike) : xbsIndex_r4/Reinterpret  force_arith_type=on  arith_type=Unsigned  force_bin_pt=off  bin_pt=0  block_type=reinterpret
  Relational{1..7} (7 alike) : xbsIndex_r4/Relational  mode=a=b  en=off  latency=0  dbl_ovrd=off  block_type=relational
  cnt{1..7} (7 alike) : xbsIndex_r4/AddSub  mode=Addition  use_carryin=off  use_carryout=off  en=off  latency=1  precision=Full  arith_type=Unsigned  n_bits=64  bin_pt=0  quantization=Truncate  overflow=Saturate  dbl_ovrd=off  use_behavioral_HDL=off  hw_selection=Fabric  pipelined=on  block_type=addsub
  sum : Outport
  wires:
  cnt7/1 -> sum/1
  cnt6/1 -> cnt7/2
  cnt3/1 -> cnt7/1
  Delay5/1 -> Delay3/1, Relational6/1
  Delay3/1 -> Delay2/1, Relational5/1
  Delay2/1 -> Delay1/1, Relational4/1
  Delay1/1 -> Delay4/1, Relational7/1
  din/1 -> Delay5/1, Relational6/2, Relational1/2, Relational2/2, Relational3/2, Relational7/2, Relational4/2, Relational5/2
  Reinterpret6/1 -> cnt4/1
  Relational7/1 -> Reinterpret6/1
  Reinterpret4/1 -> cnt5/1
  Reinterpret5/1 -> cnt5/2
  Reinterpret3/1 -> cnt4/2
  cnt4/1 -> cnt6/1
  Relational5/1 -> Reinterpret4/1
  Relational6/1 -> Reinterpret5/1
  Relational4/1 -> Reinterpret3/1
  cnt5/1 -> cnt6/2
  Delay7/1 -> Relational1/1
  Delay6/1 -> Delay7/1, Relational2/1
  Reinterpret1/1 -> cnt1/1
  Delay4/1 -> Delay6/1, Relational3/1
  Reinterpret2/1 -> cnt1/2
  Reinterpret/1 -> cnt2/2
  cnt2/1 -> cnt3/1
  Relational2/1 -> Reinterpret1/1
  Relational3/1 -> Reinterpret2/1
  Relational1/1 -> Reinterpret/1
  Constant3/1 -> cnt2/1
  cnt1/1 -> cnt3/2

== system adc5g_test/hist_0/core_d/subhist3/control ==
  sync : Inport
  bin : Inport  Port=2
  count : Inport  Port=3
  Constant1 : xbsIndex_r4/Constant  const=0  gui_display_data_type=Fixed-point  arith_type=Unsigned  n_bits=46  bin_pt=0  preci_type=Single  exp_width=8  frac_width=24  explicit_period=on  period=1  equ=P=C  opselect=C  inp2=PCIN>>17  opr=+  inp1=P  carry=CIN  dbl_ovrd=off  block_type=constant
  Counter : xbsIndex_r4/Counter  cnt_type=Count Limited  cnt_to=255  operation=Up  start_count=0  cnt_by_val=1  arith_type=Unsigned  n_bits=8  bin_pt=0  load_pin=off  rst=on  en=off  explicit_period=on  period=1  dbl_ovrd=off  use_behavioral_HDL=off  implementation=Fabric  block_type=counter
  Delay17 : xbsIndex_r4/Delay  rst=off  infoeditControl=Selection of Reset will increase slice count due to use of real FFs and instead of SRLs  en=off  latency=7  dbl_ovrd=off  reg_retiming=off  block_type=delay
  Delay2 : xbsIndex_r4/Delay  rst=off  infoeditControl=Selection of Reset will increase slice count due to use of real FFs and instead of SRLs  en=off  latency=7  dbl_ovrd=off  reg_retiming=off  block_type=delay
  Delay3 : xbsIndex_r4/Delay  rst=off  infoeditControl=Selection of Reset will increase slice count due to use of real FFs and instead of SRLs  en=off  latency=7  dbl_ovrd=off  reg_retiming=off  block_type=delay
  Delay5 : xbsIndex_r4/Delay  rst=off  infoeditControl=Selection of Reset will increase slice count due to use of real FFs and instead of SRLs  en=off  latency=1  dbl_ovrd=off  reg_retiming=off  block_type=delay
  Inverter1 : xbsIndex_r4/Inverter  en=off  latency=0  dbl_ovrd=off  block_type=inv
  Logical : xbsIndex_r4/Logical  logical_function=AND  inputs=2  en=off  latency=0  precision=Full  arith_type=Unsigned  n_bits=16  bin_pt=0  align_bp=on  dbl_ovrd=off  block_type=logical
  Mux2 : xbsIndex_r4/Mux  inputs=2  en=off  latency=0  precision=Full  arith_type=Unsigned  n_bits=16  bin_pt=14  quantization=Truncate  overflow=Wrap  dbl_ovrd=off  block_type=mux
  edge_detect : Subsystem  AncestorBlock=casper_library_misc/edge_detect  mask(edge_detect){edge=Rising, polarity=Active High, x_in=[0 0.08 0.08 0.16 0.16 0.24 0.24 0.32 0.32 0.4], y_in=[-1 -1  1  1  1  1  1  1  1  1], x_out=[0.6 0.68 0.68 0.76 0.76 0.84 0.84 0.92 0.92 1], y_out=[-1 -1  1  1 -1 -1 -1 -1 -1 -1]}
      mask prompts: edge "Edge Type"; polarity "Output Polarity"; x_in "Input X Positions"; y_in "Input Y Positions"; x_out "Output X Positions"; y_out "Output Y Positions"
      mask help: Output is active for one-cycle after each edge of the specified type (i.e. rising, falling or both).
      mask init: same as adc5g_test/ramptest_0/posedge
      settings: AttributesFormatString=|
      | rising edges
      | active high
      mask Help: Output is active for one-cycle after each edge of the specified type (i.e. rising, falling, or both).  Latency is zero.
      mask TypeOptions edge: Rising | Falling | Both
      mask TypeOptions polarity: Active High | Active Low
      mask Display:
      | plot(x_in_num,0.5+y_in_num*0.2);
      | plot(x_out_num,0.5+y_out_num*0.2);
  pulse_ext : casper_library_misc/pulse_ext  pulse_len=256
  addra : Outport
  dina : Outport  Port=2
  wea : Outport  Port=3
  addrb : Outport  Port=4
  dinb : Outport  Port=5
  web : Outport  Port=6
  wires:
  sync/1 -> Delay17/1
  Mux2/1 -> addra/1, Delay2/1
  pulse_ext/1 -> Mux2/1, wea/1, Inverter1/1
  Delay5/1 -> Mux2/2
  Delay2/1 -> addrb/1
  Constant1/1 -> dina/1
  Logical/1 -> web/1
  edge_detect/1(out) -> pulse_ext/1, Counter/1
  Counter/1 -> Mux2/3
  Delay3/1 -> Logical/2
  Inverter1/1 -> Logical/1, Delay3/1
  Delay17/1 -> edge_detect/1(in)
  bin/1 -> Delay5/1
  count/1 -> dinb/1

== system adc5g_test/hist_0/core_d/subhist3/precounter ==
  din : Inport
  Constant3 : xbsIndex_r4/Constant  const=1  gui_display_data_type=Fixed-point  arith_type=Unsigned  n_bits=1  bin_pt=0  preci_type=Single  exp_width=8  frac_width=24  explicit_period=on  period=1  equ=P=C  opselect=C  inp2=PCIN>>17  opr=+  inp1=P  carry=CIN  dbl_ovrd=off  block_type=constant
  Delay{1..7} (7 alike) : xbsIndex_r4/Delay  rst=off  infoeditControl=Selection of Reset will increase slice count due to use of real FFs and instead of SRLs  en=off  latency=1  dbl_ovrd=off  reg_retiming=off  block_type=delay
  Reinterpret{,1..6} (7 alike) : xbsIndex_r4/Reinterpret  force_arith_type=on  arith_type=Unsigned  force_bin_pt=off  bin_pt=0  block_type=reinterpret
  Relational{1..7} (7 alike) : xbsIndex_r4/Relational  mode=a=b  en=off  latency=0  dbl_ovrd=off  block_type=relational
  cnt{1..7} (7 alike) : xbsIndex_r4/AddSub  mode=Addition  use_carryin=off  use_carryout=off  en=off  latency=1  precision=Full  arith_type=Unsigned  n_bits=64  bin_pt=0  quantization=Truncate  overflow=Saturate  dbl_ovrd=off  use_behavioral_HDL=off  hw_selection=Fabric  pipelined=on  block_type=addsub
  sum : Outport
  wires:
  cnt1/1 -> cnt3/2
  Constant3/1 -> cnt2/1
  Relational1/1 -> Reinterpret/1
  Relational3/1 -> Reinterpret2/1
  Relational2/1 -> Reinterpret1/1
  cnt2/1 -> cnt3/1
  Reinterpret/1 -> cnt2/2
  Reinterpret2/1 -> cnt1/2
  Delay4/1 -> Relational3/1, Delay6/1
  Reinterpret1/1 -> cnt1/1
  Delay6/1 -> Relational2/1, Delay7/1
  Delay7/1 -> Relational1/1
  cnt5/1 -> cnt6/2
  Relational4/1 -> Reinterpret3/1
  Relational6/1 -> Reinterpret5/1
  Relational5/1 -> Reinterpret4/1
  cnt4/1 -> cnt6/1
  Reinterpret3/1 -> cnt4/2
  Reinterpret5/1 -> cnt5/2
  Reinterpret4/1 -> cnt5/1
  Relational7/1 -> Reinterpret6/1
  Reinterpret6/1 -> cnt4/1
  din/1 -> Relational5/2, Relational4/2, Relational7/2, Relational3/2, Relational2/2, Relational1/2, Relational6/2, Delay5/1
  Delay1/1 -> Relational7/1, Delay4/1
  Delay2/1 -> Relational4/1, Delay1/1
  Delay3/1 -> Relational5/1, Delay2/1
  Delay5/1 -> Relational6/1, Delay3/1
  cnt3/1 -> cnt7/1
  cnt6/1 -> cnt7/2
  cnt7/1 -> sum/1

== system adc5g_test/hist_0/count_a/mem/calc_add ==
  note: If reading/writing words
      | smaller than 32 bits,
      | change address so
      | that addressing is
      | big endian instead of
      | little, otherwise
      | pass through
  note: Use behavioural HDL
      | so that gets optimised
      | to minimum logic
  in : Inport
  add_sub : xbsIndex_r4/AddSub  mode=Subtraction  use_carryin=off  use_carryout=off  en=off  latency=0  precision=User Defined  arith_type=Unsigned  n_bits=max(1, log2(32/data_width))  bin_pt=0  quantization=Truncate  overflow=Wrap  dbl_ovrd=off  use_behavioral_HDL=on  hw_selection=Fabric  pipelined=off  block_type=addsub
  concat : xbsIndex_r4/Concat  num_inputs=2  dbl_ovrd=off  block_type=concat
  const : xbsIndex_r4/Constant  const=max(0, 32/data_width-1)  gui_display_data_type=Fixed-point  arith_type=Unsigned  n_bits=max(1, log2(32/data_width))  bin_pt=0  preci_type=Single  exp_width=8  frac_width=24  explicit_period=on  period=sample_rate  equ=P=C  opselect=C  inp2=PCIN>>17  opr=+  inp1=P  carry=CIN  dbl_ovrd=off  block_type=constant
      settings: block_version=11.4
  convert_addr : xbsIndex_r4/Convert  gui_display_data_type=Fixed-point  arith_type=Unsigned  n_bits=addr_width  bin_pt=0  float_type=Single  exp_bits=8  fraction_bits=24  quantization=Truncate  overflow=Wrap  en=off  latency=0  dbl_ovrd=off  pipeline=off  block_type=convert
      settings: xl_area=[0, 0, 0, 0, 0, 0, 0]
  lsw : xbsIndex_r4/Slice  nbits=max(1, log2(32/data_width))  boolean_output=off  mode=Lower Bit Location + Width  bit1=0  base1=MSB of Input  bit0=0  base0=LSB of Input  dbl_ovrd=off  block_type=slice
  manipulate : xbsIndex_r4/Constant  const=min(1, floor(32/data_width) - 1)  gui_display_data_type=Fixed-point  arith_type=Unsigned  n_bits=1  bin_pt=0  preci_type=Single  exp_width=8  frac_width=24  explicit_period=on  period=sample_rate  equ=P=C  opselect=C  inp2=PCIN>>17  opr=+  inp1=P  carry=CIN  dbl_ovrd=off  block_type=constant
      settings: block_version=11.4
  msw : xbsIndex_r4/Slice  nbits=addr_width - max(1, log2(32/data_width))  boolean_output=off  mode=Upper Bit Location + Width  bit1=0  base1=MSB of Input  bit0=0  base0=LSB of Input  dbl_ovrd=off  block_type=slice
  mux : xbsIndex_r4/Mux  inputs=2  en=off  latency=0  precision=Full  arith_type=Unsigned  n_bits=16  bin_pt=14  quantization=Truncate  overflow=Wrap  dbl_ovrd=off  block_type=mux
      settings: block_version=11.4
  out : Outport
  wires:
  in/1 -> convert_addr/1
  add_sub/1 -> concat/2
  msw/1 -> concat/1
  concat/1 -> mux/3
  manipulate/1 -> mux/1
  mux/1 -> out/1
  lsw/1 -> add_sub/2
  const/1 -> add_sub/1
  convert_addr/1 -> lsw/1, msw/1, mux/2
